simulink model "MX_Gateway"  (Simulink 9.0)
settings: Solver=FixedStepDiscrete  StartTime=0.0  StopTime=100  FixedStep=0.01  RelTol=1e-3
values from neighbouring files (symbols this model uses):
  ACTIVE = Bobcat.Parameter; CreateDefine( ACTIVE                         ,1                          ,'Define' ,'defines.h' )    [defines.m]
  ACTIVE_MODE_ONE = Bobcat.Parameter; CreateDefine( ACTIVE_MODE_ONE                ,19                         ,'Define' ,'defines.h' )    [defines.m]
  AUTHENTICATED = Bobcat.Parameter; CreateDefine( AUTHENTICATED                  ,1                          ,'Define' ,'defines.h' )    [defines.m]
  AUX_EMEA_CONTROLS = Bobcat.Parameter; CreateDefine( AUX_EMEA_CONTROLS  ,1   ,'Define' ,'defines.h' )    [enums.m]
  AUX_FLOW_HIGH = Bobcat.Parameter; CreateDefine( AUX_FLOW_HIGH           ,4  ,'Define' ,'defines.h' )    [enums.m]
  AUX_FLOW_INACTIVE = Bobcat.Parameter; CreateDefine( AUX_FLOW_INACTIVE       ,0  ,'Define' ,'defines.h' )    [enums.m]
  AUX_FLOW_LOW = Bobcat.Parameter; CreateDefine( AUX_FLOW_LOW            ,1  ,'Define' ,'defines.h' )    [enums.m]
  AUX_FLOW_MEDIUM = Bobcat.Parameter; CreateDefine( AUX_FLOW_MEDIUM         ,2  ,'Define' ,'defines.h' )    [enums.m]
  AUX_FLOW_STANDARD = Bobcat.Parameter; CreateDefine( AUX_FLOW_STANDARD       ,3  ,'Define' ,'defines.h' )    [enums.m]
  AUX_NON_FUNCTIONAL = Bobcat.Parameter; CreateDefine( AUX_NON_FUNCTIONAL ,255 ,'Define' ,'defines.h' )    [enums.m]
  BASE_ACTIVE = Bobcat.Parameter; CreateDefine( BASE_ACTIVE                    ,253                        ,'Define' ,'defines.h' )    [defines.m]
  BASE_DETENT = Bobcat.Parameter; CreateDefine( BASE_DETENT                    ,1                          ,'Define' ,'defines.h' )    [defines.m]
  BEEP_NONE = Bobcat.Parameter; CreateDefine( BEEP_NONE                      ,255                        ,'Define' ,'defines.h' )    [defines.m]
  CD_AIR_FILTER_DEBOUNCE_NORMAL = Bobcat.Parameter; CreateAliasCD( CD_AIR_FILTER_DEBOUNCE_NORMAL                        ,uint16(500)                ,'ConstantDataPointer' ,'CONSTANT_DATA' ,'AIR_FILTER_DEBOUNCE_NORMAL')    [cdata.m]    (also in hc12_cdata.m; m4_cdata.m)
  CD_AIR_FILTER_DEBOUNCE_NOT_CONNECTED = Bobcat.Parameter; CreateAliasCD( CD_AIR_FILTER_DEBOUNCE_NOT_CONNECTED                 ,uint16(3000)               ,'ConstantDataPointer' ,'CONSTANT_DATA' ,'AIR_FILTER_DEBOUNCE_NOT_CONNECTED')    [cdata.m]    (also in hc12_cdata.m; m4_cdata.m)
  CD_AIR_FILTER_DEBOUNCE_PLUGGED = Bobcat.Parameter; CreateAliasCD( CD_AIR_FILTER_DEBOUNCE_PLUGGED                       ,uint16(3000)               ,'ConstantDataPointer' ,'CONSTANT_DATA' ,'AIR_FILTER_DEBOUNCE_PLUGGED')    [cdata.m]    (also in hc12_cdata.m; m4_cdata.m)
  CD_AIR_FILTER_FUNCTION = Bobcat.Parameter; CreateAliasCD( CD_AIR_FILTER_FUNCTION                               ,uint8(255)                 ,'ConstantDataPointer' ,'CONSTANT_DATA' ,'AIR_FILTER_FUNCTION')    [cdata.m]    (also in hc12_cdata.m; m4_cdata.m)
  CD_AIR_FILTER_PLUGGED_WEIGHT = Bobcat.Parameter; CreateAliasCD( CD_AIR_FILTER_PLUGGED_WEIGHT                         ,uint8(2)                   ,'ConstantDataPointer' ,'CONSTANT_DATA' ,'AIR_FILTER_PLUGGED_WEIGHT')    [cdata.m]    (also in hc12_cdata.m; m4_cdata.m)
  CD_AIR_FILTER_UNPLUGGED_WEIGHT = Bobcat.Parameter; CreateAliasCD( CD_AIR_FILTER_UNPLUGGED_WEIGHT                       ,uint8(1)                   ,'ConstantDataPointer' ,'CONSTANT_DATA' ,'AIR_FILTER_UNPLUGGED_WEIGHT')    [cdata.m]    (also in hc12_cdata.m; m4_cdata.m)
  CD_AUTOIDLE_ARMBAR_DELAY = Bobcat.Parameter; CreateAliasCD( CD_AUTOIDLE_ARMBAR_DELAY                             ,uint8(2)                   ,'ConstantDataPointer' ,'CONSTANT_DATA' ,'AUTOIDLE_ARMBAR_DELAY')    [cdata.m]    (also in hc12_cdata.m; m4_cdata.m)
  CD_AUTOIDLE_FUNCTION = Bobcat.Parameter; CreateAliasCD( CD_AUTOIDLE_FUNCTION                                 ,uint8(255)                 ,'ConstantDataPointer' ,'CONSTANT_DATA' ,'AUTOIDLE_FUNCTION')    [cdata.m]    (also in hc12_cdata.m; m4_cdata.m)
  CD_AUTOIDLE_IDLE_DELAY = Bobcat.Parameter; CreateAliasCD( CD_AUTOIDLE_IDLE_DELAY                               ,uint8(40)                  ,'ConstantDataPointer' ,'CONSTANT_DATA' ,'AUTOIDLE_IDLE_DELAY')    [cdata.m]    (also in hc12_cdata.m; m4_cdata.m)
  CD_AUTOIDLE_LOW_IDLE_START = Bobcat.Parameter; CreateAliasCD( CD_AUTOIDLE_LOW_IDLE_START                           ,uint8(255)                 ,'ConstantDataPointer' ,'CONSTANT_DATA' ,'AUTOIDLE_LOW_IDLE_START')    [cdata.m]    (also in hc12_cdata.m; m4_cdata.m)
  CD_AUTOIDLE_PRESSURE_OFF = Bobcat.Parameter; CreateAliasCD( CD_AUTOIDLE_PRESSURE_OFF                             ,uint16(300)                ,'ConstantDataPointer' ,'CONSTANT_DATA' ,'AUTOIDLE_PRESSURE_OFF')    [cdata.m]    (also in hc12_cdata.m; m4_cdata.m)
  CD_AUTOIDLE_PRESSURE_ON = Bobcat.Parameter; CreateAliasCD( CD_AUTOIDLE_PRESSURE_ON                              ,uint16(270)                ,'ConstantDataPointer' ,'CONSTANT_DATA' ,'AUTOIDLE_PRESSURE_ON')    [cdata.m]    (also in hc12_cdata.m; m4_cdata.m)
  CD_AUTOSHIFT_FUNCTION = Bobcat.Parameter; CreateAliasCD( CD_AUTOSHIFT_FUNCTION                                ,uint8(255)                 ,'ConstantDataPointer' ,'CONSTANT_DATA' ,'AUTOSHIFT_FUNCTION')    [cdata.m]    (also in hc12_cdata.m; m4_cdata.m)
  CD_AUTOSHIFT_PHIGH1 = Bobcat.Parameter; CreateAliasCD( CD_AUTOSHIFT_PHIGH1                                  ,uint16(205)                ,'ConstantDataPointer' ,'CONSTANT_DATA' ,'AUTOSHIFT_PHIGH1')    [cdata.m]    (also in hc12_cdata.m; m4_cdata.m)
  CD_AUTOSHIFT_PHIGH2 = Bobcat.Parameter; CreateAliasCD( CD_AUTOSHIFT_PHIGH2                                  ,uint16(193)                ,'ConstantDataPointer' ,'CONSTANT_DATA' ,'AUTOSHIFT_PHIGH2')    [cdata.m]    (also in hc12_cdata.m; m4_cdata.m)
  CD_AUTOSHIFT_PHIGH_TEMP1 = Bobcat.Parameter; CreateAliasCD( CD_AUTOSHIFT_PHIGH_TEMP1                             ,int16(70)                  ,'ConstantDataPointer' ,'CONSTANT_DATA' ,'AUTOSHIFT_PHIGH_TEMP1')    [cdata.m]    (also in hc12_cdata.m; m4_cdata.m)
  CD_AUTOSHIFT_PHIGH_TEMP2 = Bobcat.Parameter; CreateAliasCD( CD_AUTOSHIFT_PHIGH_TEMP2                             ,int16(160)                 ,'ConstantDataPointer' ,'CONSTANT_DATA' ,'AUTOSHIFT_PHIGH_TEMP2')    [cdata.m]    (also in hc12_cdata.m; m4_cdata.m)
  CD_AUTOSHIFT_PLOW1 = Bobcat.Parameter; CreateAliasCD( CD_AUTOSHIFT_PLOW1                                   ,uint16(130)                ,'ConstantDataPointer' ,'CONSTANT_DATA' ,'AUTOSHIFT_PLOW1')    [cdata.m]    (also in hc12_cdata.m; m4_cdata.m)
  CD_AUTOSHIFT_PLOW2 = Bobcat.Parameter; CreateAliasCD( CD_AUTOSHIFT_PLOW2                                   ,uint16(100)                ,'ConstantDataPointer' ,'CONSTANT_DATA' ,'AUTOSHIFT_PLOW2')    [cdata.m]    (also in hc12_cdata.m; m4_cdata.m)
  CD_AUTOSHIFT_PLOW_TEMP1 = Bobcat.Parameter; CreateAliasCD( CD_AUTOSHIFT_PLOW_TEMP1                              ,int16(20)                  ,'ConstantDataPointer' ,'CONSTANT_DATA' ,'AUTOSHIFT_PLOW_TEMP1')    [cdata.m]    (also in hc12_cdata.m; m4_cdata.m)
  CD_AUTOSHIFT_PLOW_TEMP2 = Bobcat.Parameter; CreateAliasCD( CD_AUTOSHIFT_PLOW_TEMP2                              ,int16(80)                  ,'ConstantDataPointer' ,'CONSTANT_DATA' ,'AUTOSHIFT_PLOW_TEMP2')    [cdata.m]    (also in hc12_cdata.m; m4_cdata.m)
  CD_AUTOSHIFT_TRAVEL_DELAY = Bobcat.Parameter; CreateAliasCD( CD_AUTOSHIFT_TRAVEL_DELAY                            ,uint8(32)                  ,'ConstantDataPointer' ,'CONSTANT_DATA' ,'AUTOSHIFT_TRAVEL_DELAY')    [cdata.m]    (also in hc12_cdata.m; m4_cdata.m)
  CD_AUX2_CRACKPOINT_CURRENT = Bobcat.Parameter; CreateAliasCD( CD_AUX2_CRACKPOINT_CURRENT                           ,fi(0,0,16,12,'hex','0c28') ,'ConstantDataPointer' ,'CONSTANT_DATA' ,'AUX2_CRACKPOINT_CURRENT')    [cdata.m]    (also in hc12_cdata.m; m4_cdata.m)
  CD_AUX2_FLOW_LOW_FUNCTION = Bobcat.Parameter; CreateAliasCD( CD_AUX2_FLOW_LOW_FUNCTION                            ,uint8(255)                 ,'ConstantDataPointer' ,'CONSTANT_DATA' ,'AUX2_FLOW_LOW_FUNCTION')    [cdata.m]    (also in hc12_cdata.m; m4_cdata.m)
  CD_AUX2_FLOW_MED_FUNCTION = Bobcat.Parameter; CreateAliasCD( CD_AUX2_FLOW_MED_FUNCTION                            ,uint8(255)                 ,'ConstantDataPointer' ,'CONSTANT_DATA' ,'AUX2_FLOW_MED_FUNCTION')    [cdata.m]    (also in hc12_cdata.m; m4_cdata.m)
  CD_AUX2_HI_FLOW_PERCENT = Bobcat.Parameter; CreateAliasCD( CD_AUX2_HI_FLOW_PERCENT                              ,fi(0,0,16,16,'hex','ffff') ,'ConstantDataPointer' ,'CONSTANT_DATA' ,'AUX2_HI_FLOW_PERCENT')    [cdata.m]    (also in hc12_cdata.m; m4_cdata.m)
  CD_AUX2_LOW_FLOW_PERCENT = Bobcat.Parameter; CreateAliasCD( CD_AUX2_LOW_FLOW_PERCENT                             ,fi(0,0,16,16,'hex','7333') ,'ConstantDataPointer' ,'CONSTANT_DATA' ,'AUX2_LOW_FLOW_PERCENT')    [cdata.m]    (also in hc12_cdata.m; m4_cdata.m)
  CD_AUX2_MAX_FLOW_CURRENT = Bobcat.Parameter; CreateAliasCD( CD_AUX2_MAX_FLOW_CURRENT                             ,fi(0,0,16,12,'hex','14cc') ,'ConstantDataPointer' ,'CONSTANT_DATA' ,'AUX2_MAX_FLOW_CURRENT')    [cdata.m]    (also in hc12_cdata.m; m4_cdata.m)
  CD_AUX2_MED_FLOW_PERCENT = Bobcat.Parameter; CreateAliasCD( CD_AUX2_MED_FLOW_PERCENT                             ,fi(0,0,16,16,'hex','9999') ,'ConstantDataPointer' ,'CONSTANT_DATA' ,'AUX2_MED_FLOW_PERCENT')    [cdata.m]    (also in hc12_cdata.m; m4_cdata.m)
  CD_AUX_CRACKPOINT_CURRENT = Bobcat.Parameter; CreateAliasCD( CD_AUX_CRACKPOINT_CURRENT                            ,fi(0,0,16,12,'hex','0000') ,'ConstantDataPointer' ,'CONSTANT_DATA' ,'AUX_CRACKPOINT_CURRENT')    [cdata.m]    (also in hc12_cdata.m; m4_cdata.m)
  CD_AUX_DETENT_FLASH_TIME = Bobcat.Parameter; CreateAliasCD( CD_AUX_DETENT_FLASH_TIME                             ,uint16(72)                 ,'ConstantDataPointer' ,'CONSTANT_DATA' ,'AUX_DETENT_FLASH_TIME')    [cdata.m]    (also in hc12_cdata.m; m4_cdata.m)
  CD_AUX_DETENT_HOLD_TIME = Bobcat.Parameter; CreateAliasCD( CD_AUX_DETENT_HOLD_TIME                              ,uint16(100)                ,'ConstantDataPointer' ,'CONSTANT_DATA' ,'AUX_DETENT_HOLD_TIME')    [cdata.m]    (also in hc12_cdata.m; m4_cdata.m)
  CD_AUX_FULL_ON_AUX1 = Bobcat.Parameter; CreateAliasCD( CD_AUX_FULL_ON_AUX1                                  ,fi(0,0,16,12,'hex','10cd') ,'ConstantDataPointer' ,'CONSTANT_DATA' ,'AUX_FULL_ON_AUX1')    [cdata.m]    (also in hc12_cdata.m; m4_cdata.m)
  CD_AUX_FULL_ON_AUX2 = Bobcat.Parameter; CreateAliasCD( CD_AUX_FULL_ON_AUX2                                  ,fi(0,0,16,12,'hex','139d') ,'ConstantDataPointer' ,'CONSTANT_DATA' ,'AUX_FULL_ON_AUX2')    [cdata.m]    (also in hc12_cdata.m; m4_cdata.m)
  CD_AUX_FULL_ON_AUX3 = Bobcat.Parameter; CreateAliasCD( CD_AUX_FULL_ON_AUX3                                  ,fi(0,0,16,12,'hex','17d7') ,'ConstantDataPointer' ,'CONSTANT_DATA' ,'AUX_FULL_ON_AUX3')    [cdata.m]    (also in hc12_cdata.m; m4_cdata.m)
  CD_AUX_FUNCTION = Bobcat.Parameter; CreateAliasCD( CD_AUX_FUNCTION                                      ,uint8(0)                   ,'ConstantDataPointer' ,'CONSTANT_DATA' ,'AUX_FUNCTION')    [cdata.m]    (also in hc12_cdata.m; m4_cdata.m)
  CD_AUX_RATE_LIMIT_CRACKPOINT = Bobcat.Parameter; CreateAliasCD( CD_AUX_RATE_LIMIT_CRACKPOINT                         ,fi(0,0,16,12,'hex','0800') ,'ConstantDataPointer' ,'CONSTANT_DATA' ,'AUX_RATE_LIMIT_CRACKPOINT')    [cdata.m]    (also in hc12_cdata.m; m4_cdata.m)
  CD_AUX_RATE_LIMIT_CRACKPOINT_RATE = Bobcat.Parameter; CreateAliasCD( CD_AUX_RATE_LIMIT_CRACKPOINT_RATE                    ,fi(0,1,32,12,'int',102400) ,'ConstantDataPointer' ,'CONSTANT_DATA' ,'AUX_RATE_LIMIT_CRACKPOINT_RATE')    [cdata.m]    (also in hc12_cdata.m; m4_cdata.m)
  CD_AUX_RATE_LIMIT_FUNCTION = Bobcat.Parameter; CreateAliasCD( CD_AUX_RATE_LIMIT_FUNCTION                           ,uint8(255)                 ,'ConstantDataPointer' ,'CONSTANT_DATA' ,'AUX_RATE_LIMIT_FUNCTION')    [cdata.m]    (also in hc12_cdata.m; m4_cdata.m)
  CD_AUX_RATE_LIMIT_MAX_ENG_SPD = Bobcat.Parameter; CreateAliasCD( CD_AUX_RATE_LIMIT_MAX_ENG_SPD                        ,uint16(1600)               ,'ConstantDataPointer' ,'CONSTANT_DATA' ,'AUX_RATE_LIMIT_MAX_ENG_SPD')    [cdata.m]    (also in hc12_cdata.m; m4_cdata.m)
  CD_AUX_RATE_LIMIT_MIN_ENG_SPD = Bobcat.Parameter; CreateAliasCD( CD_AUX_RATE_LIMIT_MIN_ENG_SPD                        ,uint16(100)                ,'ConstantDataPointer' ,'CONSTANT_DATA' ,'AUX_RATE_LIMIT_MIN_ENG_SPD')    [cdata.m]    (also in hc12_cdata.m; m4_cdata.m)
  CD_AUX_RATE_LIMIT_NORMAL_RATE = Bobcat.Parameter; CreateAliasCD( CD_AUX_RATE_LIMIT_NORMAL_RATE                        ,fi(0,1,32,12,'int',2048)   ,'ConstantDataPointer' ,'CONSTANT_DATA' ,'AUX_RATE_LIMIT_NORMAL_RATE')    [cdata.m]    (also in hc12_cdata.m; m4_cdata.m)
  CD_AUXPOT_CAL_DEADBAND_HIGH = Bobcat.Parameter; CreateAliasCD( CD_AUXPOT_CAL_DEADBAND_HIGH                          ,uint16(574)                ,'ConstantDataPointer' ,'CONSTANT_DATA' ,'AUXPOT_CAL_DEADBAND_HIGH')    [cdata.m]    (also in hc12_cdata.m; m4_cdata.m)
  CD_AUXPOT_CAL_DEADBAND_LOW = Bobcat.Parameter; CreateAliasCD( CD_AUXPOT_CAL_DEADBAND_LOW                           ,uint16(424)                ,'ConstantDataPointer' ,'CONSTANT_DATA' ,'AUXPOT_CAL_DEADBAND_LOW')    [cdata.m]    (also in hc12_cdata.m; m4_cdata.m)
  CD_AUXPOT_CENTER_RATIO = Bobcat.Parameter; CreateAliasCD( CD_AUXPOT_CENTER_RATIO                               ,uint16(500)                ,'ConstantDataPointer' ,'CONSTANT_DATA' ,'AUXPOT_CENTER_RATIO')    [cdata.m]    (also in hc12_cdata.m; m4_cdata.m)
  CD_AUXPOT_DEADBAND = Bobcat.Parameter; CreateAliasCD( CD_AUXPOT_DEADBAND                                   ,uint8(38)                  ,'ConstantDataPointer' ,'CONSTANT_DATA' ,'AUXPOT_DEADBAND')    [cdata.m]    (also in hc12_cdata.m; m4_cdata.m)
  CD_AUXPOT_FUNCTION = Bobcat.Parameter; CreateAliasCD( CD_AUXPOT_FUNCTION                                   ,uint8(0)                   ,'ConstantDataPointer' ,'CONSTANT_DATA' ,'AUXPOT_FUNCTION')    [cdata.m]    (also in hc12_cdata.m; m4_cdata.m)
  CD_AUXPOT_RATIO_GAIN = Bobcat.Parameter; CreateAliasCD( CD_AUXPOT_RATIO_GAIN                                 ,uint16(1000)               ,'ConstantDataPointer' ,'CONSTANT_DATA' ,'AUXPOT_RATIO_GAIN')    [cdata.m]    (also in hc12_cdata.m; m4_cdata.m)
  CD_CAN_THROTTLE_FUNCTION = Bobcat.Parameter; CreateAliasCD( CD_CAN_THROTTLE_FUNCTION                             ,uint8(255)                 ,'ConstantDataPointer' ,'CONSTANT_DATA' ,'CAN_THROTTLE_FUNCTION')    [cdata.m]    (also in hc12_cdata.m; m4_cdata.m)
  CD_CAN_THROTTLE_REVERSE = Bobcat.Parameter; CreateAliasCD( CD_CAN_THROTTLE_REVERSE                              ,uint8(255)                 ,'ConstantDataPointer' ,'CONSTANT_DATA' ,'CAN_THROTTLE_REVERSE')    [cdata.m]    (also in hc12_cdata.m; m4_cdata.m)
  CD_CONSOLE_DEBOUNCE_ABSENT = Bobcat.Parameter; CreateAliasCD( CD_CONSOLE_DEBOUNCE_ABSENT                           ,uint16(12)                 ,'ConstantDataPointer' ,'CONSTANT_DATA' ,'CONSOLE_DEBOUNCE_ABSENT')    [cdata.m]    (also in hc12_cdata.m; m4_cdata.m)
  CD_CONSOLE_DEBOUNCE_OORH = Bobcat.Parameter; CreateAliasCD( CD_CONSOLE_DEBOUNCE_OORH                             ,uint16(12)                 ,'ConstantDataPointer' ,'CONSTANT_DATA' ,'CONSOLE_DEBOUNCE_OORH')    [cdata.m]    (also in hc12_cdata.m; m4_cdata.m)
  CD_CONSOLE_DEBOUNCE_OORL = Bobcat.Parameter; CreateAliasCD( CD_CONSOLE_DEBOUNCE_OORL                             ,uint16(12)                 ,'ConstantDataPointer' ,'CONSTANT_DATA' ,'CONSOLE_DEBOUNCE_OORL')    [cdata.m]    (also in hc12_cdata.m; m4_cdata.m)
  CD_CONSOLE_DEBOUNCE_PRESENT = Bobcat.Parameter; CreateAliasCD( CD_CONSOLE_DEBOUNCE_PRESENT                          ,uint16(12)                 ,'ConstantDataPointer' ,'CONSTANT_DATA' ,'CONSOLE_DEBOUNCE_PRESENT')    [cdata.m]    (also in hc12_cdata.m; m4_cdata.m)
  CD_CONSOLE_FUNCTION = Bobcat.Parameter; CreateAliasCD( CD_CONSOLE_FUNCTION                                  ,uint8(0)                   ,'ConstantDataPointer' ,'CONSTANT_DATA' ,'CONSOLE_FUNCTION')    [cdata.m]    (also in hc12_cdata.m; m4_cdata.m)
  CD_CONSOLE_OORH = Bobcat.Parameter; CreateAliasCD( CD_CONSOLE_OORH                                      ,uint16(740)                ,'ConstantDataPointer' ,'CONSTANT_DATA' ,'CONSOLE_OORH')    [cdata.m]    (also in hc12_cdata.m; m4_cdata.m)
  CD_CONSOLE_OORL = Bobcat.Parameter; CreateAliasCD( CD_CONSOLE_OORL                                      ,uint16(10)                 ,'ConstantDataPointer' ,'CONSTANT_DATA' ,'CONSOLE_OORL')    [cdata.m]    (also in hc12_cdata.m; m4_cdata.m)
  CD_CONSOLE_PRESENT_HIGH = Bobcat.Parameter; CreateAliasCD( CD_CONSOLE_PRESENT_HIGH                              ,uint16(515)                ,'ConstantDataPointer' ,'CONSTANT_DATA' ,'CONSOLE_PRESENT_HIGH')    [cdata.m]    (also in hc12_cdata.m; m4_cdata.m)
  CD_CONSOLE_PRESENT_LOW = Bobcat.Parameter; CreateAliasCD( CD_CONSOLE_PRESENT_LOW                               ,uint16(375)                ,'ConstantDataPointer' ,'CONSTANT_DATA' ,'CONSOLE_PRESENT_LOW')    [cdata.m]    (also in hc12_cdata.m; m4_cdata.m)
  CD_DELUXE_GEN5_5_FUNCTION = Bobcat.Parameter; CreateAliasCD( CD_DELUXE_GEN5_5_FUNCTION                            ,uint8(255)                 ,'ConstantDataPointer' ,'CONSTANT_DATA' ,'DELUXE_GEN5_5_FUNCTION')    [cdata.m]    (also in hc12_cdata.m; m4_cdata.m)
  CD_DELUXE_GEN5_FUNCTION = Bobcat.Parameter; CreateAliasCD( CD_DELUXE_GEN5_FUNCTION                              ,uint8(255)                 ,'ConstantDataPointer' ,'CONSTANT_DATA' ,'DELUXE_GEN5_FUNCTION')    [cdata.m]    (also in hc12_cdata.m; m4_cdata.m)
  CD_DERATE_ENGINE_SPEED_DEBOUNCE_HIGH = Bobcat.Parameter; CreateAliasCD( CD_DERATE_ENGINE_SPEED_DEBOUNCE_HIGH                 ,uint16(200)                ,'ConstantDataPointer' ,'CONSTANT_DATA' ,'DERATE_ENGINE_SPEED_DEBOUNCE_HIGH')    [cdata.m]    (also in hc12_cdata.m; m4_cdata.m)
  CD_DERATE_ENGINE_SPEED_DEBOUNCE_SHUTDOWN = Bobcat.Parameter; CreateAliasCD( CD_DERATE_ENGINE_SPEED_DEBOUNCE_SHUTDOWN             ,uint16(1000)               ,'ConstantDataPointer' ,'CONSTANT_DATA' ,'DERATE_ENGINE_SPEED_DEBOUNCE_SHUTDOWN')    [cdata.m]    (also in hc12_cdata.m; m4_cdata.m)
  CD_DERATE_ENGINE_SPEED_HIGH_OFFSET = Bobcat.Parameter; CreateAliasCD( CD_DERATE_ENGINE_SPEED_HIGH_OFFSET                   ,uint16(200)                ,'ConstantDataPointer' ,'CONSTANT_DATA' ,'DERATE_ENGINE_SPEED_HIGH_OFFSET')    [cdata.m]    (also in hc12_cdata.m; m4_cdata.m)
  CD_DERATE_METHOD = Bobcat.Parameter; CreateAliasCD( CD_DERATE_METHOD                                     ,uint8(0)                   ,'ConstantDataPointer' ,'CONSTANT_DATA' ,'DERATE_METHOD')    [cdata.m]    (also in hc12_cdata.m; m4_cdata.m)
  CD_DUMP_VALVE_FUNCTION = Bobcat.Parameter; CreateAliasCD( CD_DUMP_VALVE_FUNCTION                               ,uint8(255)                 ,'ConstantDataPointer' ,'CONSTANT_DATA' ,'DUMP_VALVE_FUNCTION')    [cdata.m]    (also in hc12_cdata.m; m4_cdata.m)
  CD_DUMP_VALVE_ON_CURRENT = Bobcat.Parameter; CreateAliasCD( CD_DUMP_VALVE_ON_CURRENT                             ,fi(0,0,16,12,'hex','ffff') ,'ConstantDataPointer' ,'CONSTANT_DATA' ,'DUMP_VALVE_ON_CURRENT')    [cdata.m]    (also in hc12_cdata.m; m4_cdata.m)
  CD_ECO_MODE_THROTTLE_REDUCTION = Bobcat.Parameter; CreateAliasCD( CD_ECO_MODE_THROTTLE_REDUCTION                       ,uint16(850)                ,'ConstantDataPointer' ,'CONSTANT_DATA' ,'ECO_MODE_THROTTLE_REDUCTION')    [cdata.m]    (also in hc12_cdata.m; m4_cdata.m)
  CD_ECT_FILTER_GAIN = Bobcat.Parameter; CreateAliasCD( CD_ECT_FILTER_GAIN                                   ,uint8(6)                   ,'ConstantDataPointer' ,'CONSTANT_DATA' ,'ECT_FILTER_GAIN')    [cdata.m]    (also in hc12_cdata.m; m4_cdata.m)
  CD_ECU_FUNCTION = Bobcat.Parameter; CreateAliasCD( CD_ECU_FUNCTION                                      ,uint8(255)                 ,'ConstantDataPointer' ,'CONSTANT_DATA' ,'ECU_FUNCTION')    [cdata.m]    (also in hc12_cdata.m; m4_cdata.m)
  CD_ECU_HOLD_DEBOUNCE_ERROR_ON = Bobcat.Parameter; CreateAliasCD( CD_ECU_HOLD_DEBOUNCE_ERROR_ON                        ,uint16(20)                 ,'ConstantDataPointer' ,'CONSTANT_DATA' ,'ECU_HOLD_DEBOUNCE_ERROR_ON')    [cdata.m]    (also in hc12_cdata.m; m4_cdata.m)
  CD_EMEA_CONTROLS_HOLD_TIME = Bobcat.Parameter; CreateAliasCD( CD_EMEA_CONTROLS_HOLD_TIME                           ,uint16(30)                 ,'ConstantDataPointer' ,'CONSTANT_DATA' ,'EMEA_CONTROLS_HOLD_TIME')    [cdata.m]    (also in hc12_cdata.m; m4_cdata.m)
  CD_EMEA_CONTROLS_OPTION_A = Bobcat.Parameter; CreateAliasCD( CD_EMEA_CONTROLS_OPTION_A                            ,uint8(255)                 ,'ConstantDataPointer' ,'CONSTANT_DATA' ,'EMEA_CONTROLS_OPTION_A')    [cdata.m]    (also in hc12_cdata.m; m4_cdata.m)
  CD_EMEA_CONTROLS_OPTION_C = Bobcat.Parameter; CreateAliasCD( CD_EMEA_CONTROLS_OPTION_C                            ,uint8(255)                 ,'ConstantDataPointer' ,'CONSTANT_DATA' ,'EMEA_CONTROLS_OPTION_C')    [cdata.m]    (also in hc12_cdata.m; m4_cdata.m)
  CD_EMEA_CONTROLS_REVERSE = Bobcat.Parameter; CreateAliasCD( CD_EMEA_CONTROLS_REVERSE                             ,uint8(255)                 ,'ConstantDataPointer' ,'CONSTANT_DATA' ,'EMEA_CONTROLS_REVERSE')    [cdata.m]    (also in hc12_cdata.m; m4_cdata.m)
  CD_EMEA_ELECTRIC_VERSION = Bobcat.Parameter; CreateAliasCD( CD_EMEA_ELECTRIC_VERSION                             ,uint8(0)                   ,'ConstantDataPointer' ,'CONSTANT_DATA' ,'EMEA_ELECTRIC_VERSION')    [cdata.m]    (also in hc12_cdata.m; m4_cdata.m)
  CD_EMEA_POT_CAL_DEADBAND_HIGH = Bobcat.Parameter; CreateAliasCD( CD_EMEA_POT_CAL_DEADBAND_HIGH                        ,uint16(574)                ,'ConstantDataPointer' ,'CONSTANT_DATA' ,'EMEA_POT_CAL_DEADBAND_HIGH')    [cdata.m]    (also in hc12_cdata.m; m4_cdata.m)
  CD_EMEA_POT_CAL_DEADBAND_LOW = Bobcat.Parameter; CreateAliasCD( CD_EMEA_POT_CAL_DEADBAND_LOW                         ,uint16(424)                ,'ConstantDataPointer' ,'CONSTANT_DATA' ,'EMEA_POT_CAL_DEADBAND_LOW')    [cdata.m]    (also in hc12_cdata.m; m4_cdata.m)
  CD_ENGINE_RPM_FACTOR = Bobcat.Parameter; CreateAliasCD( CD_ENGINE_RPM_FACTOR                                 ,uint8(35)                  ,'ConstantDataPointer' ,'CONSTANT_DATA' ,'ENGINE_RPM_FACTOR')    [cdata.m]    (also in hc12_cdata.m; m4_cdata.m)
  CD_ENGINE_SPEED_FILTER_FAST_VALUE = Bobcat.Parameter; CreateAliasCD( CD_ENGINE_SPEED_FILTER_FAST_VALUE                    ,uint8(1)                   ,'ConstantDataPointer' ,'CONSTANT_DATA' ,'ENGINE_SPEED_FILTER_FAST_VALUE')    [cdata.m]    (also in hc12_cdata.m; m4_cdata.m)
  CD_ENGINE_SPEED_FILTER_SLOW_VALUE = Bobcat.Parameter; CreateAliasCD( CD_ENGINE_SPEED_FILTER_SLOW_VALUE                    ,uint8(4)                   ,'ConstantDataPointer' ,'CONSTANT_DATA' ,'ENGINE_SPEED_FILTER_SLOW_VALUE')    [cdata.m]    (also in hc12_cdata.m; m4_cdata.m)
  CD_ENGINE_SPEED_RUNNING = Bobcat.Parameter; CreateAliasCD( CD_ENGINE_SPEED_RUNNING                              ,uint16(800)                ,'ConstantDataPointer' ,'CONSTANT_DATA' ,'ENGINE_SPEED_RUNNING')    [cdata.m]    (also in hc12_cdata.m; m4_cdata.m)
  CD_ENGINE_START_DELAY_DEBOUNCE_RPM_NO_SIGNAL = Bobcat.Parameter; CreateAliasCD( CD_ENGINE_START_DELAY_DEBOUNCE_RPM_NO_SIGNAL         ,uint16(0)                  ,'ConstantDataPointer' ,'CONSTANT_DATA' ,'ENGINE_START_DELAY_DEBOUNCE_RPM_NO_SIGNAL')    [cdata.m]    (also in hc12_cdata.m; m4_cdata.m)
  CD_ENGINE_START_DELAY_OFF_TIME = Bobcat.Parameter; CreateAliasCD( CD_ENGINE_START_DELAY_OFF_TIME                       ,uint16(0)                  ,'ConstantDataPointer' ,'CONSTANT_DATA' ,'ENGINE_START_DELAY_OFF_TIME')    [cdata.m]    (also in hc12_cdata.m; m4_cdata.m)
  CD_ENGINE_START_DELAY_RPM_NO_SIGNAL = Bobcat.Parameter; CreateAliasCD( CD_ENGINE_START_DELAY_RPM_NO_SIGNAL                  ,uint16(0)                  ,'ConstantDataPointer' ,'CONSTANT_DATA' ,'ENGINE_START_DELAY_RPM_NO_SIGNAL')    [cdata.m]    (also in hc12_cdata.m; m4_cdata.m)
  CD_EOP_DEBOUNCE_EXTREMELY_LOW = Bobcat.Parameter; CreateAliasCD( CD_EOP_DEBOUNCE_EXTREMELY_LOW                        ,uint16(50)                 ,'ConstantDataPointer' ,'CONSTANT_DATA' ,'EOP_DEBOUNCE_EXTREMELY_LOW')    [cdata.m]    (also in hc12_cdata.m; m4_cdata.m)
  CD_EOP_DEBOUNCE_NORMAL = Bobcat.Parameter; CreateAliasCD( CD_EOP_DEBOUNCE_NORMAL                               ,uint16(50)                 ,'ConstantDataPointer' ,'CONSTANT_DATA' ,'EOP_DEBOUNCE_NORMAL')    [cdata.m]    (also in hc12_cdata.m; m4_cdata.m)
  CD_EOP_DEBOUNCE_SHUTDOWN = Bobcat.Parameter; CreateAliasCD( CD_EOP_DEBOUNCE_SHUTDOWN                             ,uint16(100)                ,'ConstantDataPointer' ,'CONSTANT_DATA' ,'EOP_DEBOUNCE_SHUTDOWN')    [cdata.m]    (also in hc12_cdata.m; m4_cdata.m)
  CD_EOP_FILTER_VALUE = Bobcat.Parameter; CreateAliasCD( CD_EOP_FILTER_VALUE                                  ,uint8(3)                   ,'ConstantDataPointer' ,'CONSTANT_DATA' ,'EOP_FILTER_VALUE')    [cdata.m]    (also in hc12_cdata.m; m4_cdata.m)
  CD_EOP_FUNCTION = Bobcat.Parameter; CreateAliasCD( CD_EOP_FUNCTION                                      ,uint8(1)                   ,'ConstantDataPointer' ,'CONSTANT_DATA' ,'EOP_FUNCTION')    [cdata.m]    (also in hc12_cdata.m; m4_cdata.m)
  CD_EOP_SWITCH_GAUGE_VALUE = Bobcat.Parameter; CreateAliasCD( CD_EOP_SWITCH_GAUGE_VALUE                            ,uint16(125)                ,'ConstantDataPointer' ,'CONSTANT_DATA' ,'EOP_SWITCH_GAUGE_VALUE')    [cdata.m]    (also in hc12_cdata.m; m4_cdata.m)
  CD_EOP_SWITCH_LOW = Bobcat.Parameter; CreateAliasCD( CD_EOP_SWITCH_LOW                                    ,uint16(50)                 ,'ConstantDataPointer' ,'CONSTANT_DATA' ,'EOP_SWITCH_LOW')    [cdata.m]    (also in hc12_cdata.m; m4_cdata.m)
  CD_EOP_SWITCH_PRESSURE_VALUE = Bobcat.Parameter; CreateAliasCD( CD_EOP_SWITCH_PRESSURE_VALUE                         ,uint16(40)                 ,'ConstantDataPointer' ,'CONSTANT_DATA' ,'EOP_SWITCH_PRESSURE_VALUE')    [cdata.m]    (also in hc12_cdata.m; m4_cdata.m)
  CD_EOT_DEBOUNCE_EXTREMELY_LOW = Bobcat.Parameter; CreateAliasCD( CD_EOT_DEBOUNCE_EXTREMELY_LOW                        ,uint16(2)                  ,'ConstantDataPointer' ,'CONSTANT_DATA' ,'EOT_DEBOUNCE_EXTREMELY_LOW')    [cdata.m]    (also in hc12_cdata.m; m4_cdata.m)
  CD_EOT_DEBOUNCE_LOW = Bobcat.Parameter; CreateAliasCD( CD_EOT_DEBOUNCE_LOW                                  ,uint16(2)                  ,'ConstantDataPointer' ,'CONSTANT_DATA' ,'EOT_DEBOUNCE_LOW')    [cdata.m]    (also in hc12_cdata.m; m4_cdata.m)
  CD_EOT_DEBOUNCE_NORMAL = Bobcat.Parameter; CreateAliasCD( CD_EOT_DEBOUNCE_NORMAL                               ,uint16(2)                  ,'ConstantDataPointer' ,'CONSTANT_DATA' ,'EOT_DEBOUNCE_NORMAL')    [cdata.m]    (also in hc12_cdata.m; m4_cdata.m)
  CD_EOT_EXTREMELY_LOW = Bobcat.Parameter; CreateAliasCD( CD_EOT_EXTREMELY_LOW                                 ,uint16(81)                 ,'ConstantDataPointer' ,'CONSTANT_DATA' ,'EOT_EXTREMELY_LOW')    [cdata.m]    (also in hc12_cdata.m; m4_cdata.m)
  CD_EOT_FUNCTION = Bobcat.Parameter; CreateAliasCD( CD_EOT_FUNCTION                                      ,uint8(255)                 ,'ConstantDataPointer' ,'CONSTANT_DATA' ,'EOT_FUNCTION')    [cdata.m]    (also in hc12_cdata.m; m4_cdata.m)
  CD_EOT_LOW = Bobcat.Parameter; CreateAliasCD( CD_EOT_LOW                                           ,uint16(90)                 ,'ConstantDataPointer' ,'CONSTANT_DATA' ,'EOT_LOW')    [cdata.m]    (also in hc12_cdata.m; m4_cdata.m)
  CD_EOT_TEMPERATURE_HYSTERESIS = Bobcat.Parameter; CreateAliasCD( CD_EOT_TEMPERATURE_HYSTERESIS                        ,uint16(2)                  ,'ConstantDataPointer' ,'CONSTANT_DATA' ,'EOT_TEMPERATURE_HYSTERESIS')    [cdata.m]    (also in hc12_cdata.m; m4_cdata.m)
  CD_FAST_TRAVEL_FUNCTION = Bobcat.Parameter; CreateAliasCD( CD_FAST_TRAVEL_FUNCTION                              ,uint8(255)                 ,'ConstantDataPointer' ,'CONSTANT_DATA' ,'FAST_TRAVEL_FUNCTION')    [cdata.m]    (also in hc12_cdata.m; m4_cdata.m)
  CD_FAST_TRAVEL_THROTTLE_DELAY = Bobcat.Parameter; CreateAliasCD( CD_FAST_TRAVEL_THROTTLE_DELAY                        ,uint16(10)                 ,'ConstantDataPointer' ,'CONSTANT_DATA' ,'FAST_TRAVEL_THROTTLE_DELAY')    [cdata.m]    (also in hc12_cdata.m; m4_cdata.m)
  CD_FAST_TRAVEL_THROTTLE_LIMIT = Bobcat.Parameter; CreateAliasCD( CD_FAST_TRAVEL_THROTTLE_LIMIT                        ,uint16(1000)               ,'ConstantDataPointer' ,'CONSTANT_DATA' ,'FAST_TRAVEL_THROTTLE_LIMIT')    [cdata.m]    (also in hc12_cdata.m; m4_cdata.m)
  CD_FLYWHEEL_TEETH = Bobcat.Parameter; CreateAliasCD( CD_FLYWHEEL_TEETH                                    ,uint8(83)                  ,'ConstantDataPointer' ,'CONSTANT_DATA' ,'FLYWHEEL_TEETH')    [cdata.m]    (also in hc12_cdata.m; m4_cdata.m)
  CD_FUEL_LEVEL_FILTER_FAST_VALUE = Bobcat.Parameter; CreateAliasCD( CD_FUEL_LEVEL_FILTER_FAST_VALUE                      ,uint8(5)                   ,'ConstantDataPointer' ,'CONSTANT_DATA' ,'FUEL_LEVEL_FILTER_FAST_VALUE')    [cdata.m]    (also in hc12_cdata.m; m4_cdata.m)
  CD_FUEL_LEVEL_FILTER_SLOW_VALUE = Bobcat.Parameter; CreateAliasCD( CD_FUEL_LEVEL_FILTER_SLOW_VALUE                      ,uint8(10)                  ,'ConstantDataPointer' ,'CONSTANT_DATA' ,'FUEL_LEVEL_FILTER_SLOW_VALUE')    [cdata.m]    (also in hc12_cdata.m; m4_cdata.m)
  CD_FUEL_PRIMARY_FEEDBACK_FUNCTION = Bobcat.Parameter; CreateAliasCD( CD_FUEL_PRIMARY_FEEDBACK_FUNCTION                    ,uint8(0)                   ,'ConstantDataPointer' ,'CONSTANT_DATA' ,'FUEL_PRIMARY_FEEDBACK_FUNCTION')    [cdata.m]    (also in hc12_cdata.m; m4_cdata.m)
  ... (+246 more)
undefined symbols (not defined in the file or in its folder): sigbuilder_block
undefined symbols (not defined in the file): CD_FUEL_RESOLUTION_TIMER
libraries: simulink x186, MX_Library x107
(part 44 of 48)

== stateflow chart MX_Gtwy_Control/PanelInterface/mod_HydraulicIcon/HydraulicIcon ==
  settings: executeAtInitialization=1
  state INACTIVE
    en:icon_Hydraulic = INACTIVE;
  state ERROR
    en:icon_Hydraulic = ERROR_FLASH;
  state ACTIVE
    en:icon_Hydraulic = ACTIVE;
  (default) -> INACTIVE
  INACTIVE -> ERROR [order 1] : [(state_HOT == EXTREMELY_HIGH)...
      | || (state_HOT == SHUTDOWN)]
  ERROR -> INACTIVE : [(state_HOT == NORMAL) || (state_HOT == HIGH) || (state_HOT == COLD)]
  INACTIVE -> ACTIVE [order 2] : [(state_HOT == HIGH)...
      | || (state_HOT == OORL)...
      | || (state_HOT == OORH)]
  ACTIVE -> INACTIVE [order 1] : [(state_HOT == NORMAL) || (state_HOT == COLD)]
  ACTIVE -> ERROR [order 2] : [ (state_HOT == EXTREMELY_HIGH)...
      | || (state_HOT == SHUTDOWN)]
  data: icon_Hydraulic output uint8; state_HOT input uint8; ACTIVE parameter uint8; COLD parameter uint8; EXTREMELY_HIGH parameter uint8; HIGH parameter uint8; INACTIVE parameter uint8; NORMAL parameter uint8; SHUTDOWN parameter uint8; OORL parameter uint8; OORH parameter uint8; ERROR_FLASH parameter uint8

== matlab function MX_Gtwy_Control/PanelInterface/mod_JobClock1Icon/Truth_Table ==
  data: u input uint8; y output uint8
function y = fcn(u)

aVarTruthTableCondition_1 = false;


% Job clock 1 is active

aVarTruthTableCondition_1 = logical(u == 6);

if (aVarTruthTableCondition_1)
    aFcnTruthTableAction_1();
else % Default
    aFcnTruthTableAction_2();
end

function aFcnTruthTableAction_1()

% Turn on icon.

y = uint8(1);

function aFcnTruthTableAction_2()

% Turn off icon.

y = uint8(0);

== matlab function MX_Gtwy_Control/PanelInterface/mod_JobClock2Icon/Truth_Table ==
  data: u input uint8; y output uint8
function y = fcn(u)

aVarTruthTableCondition_1 = false;


% Job clock 2 is active

aVarTruthTableCondition_1 = logical(u == 7);

if (aVarTruthTableCondition_1)
    aFcnTruthTableAction_1();
else % Default
    aFcnTruthTableAction_2();
end

function aFcnTruthTableAction_1()

% Turn on icon.

y = uint8(1);

function aFcnTruthTableAction_2()

% Turn off icon.

y = uint8(0);

== stateflow chart MX_Gtwy_Control/PanelInterface/mod_RPMIcon/RPM_ICON ==
  settings: executeAtInitialization=1
  state ERROR
    en:icon_RPM = ERROR_FLASH;
  state INACTIVE
    en:icon_RPM = INACTIVE;
  state ACTIVE
    en:icon_RPM = ACTIVE;
  (default) -> INACTIVE
  INACTIVE -> ERROR [order 1] : [(state_EngineSpeed != NORMAL)]
  ERROR -> INACTIVE : [(state_EngineSpeed == NORMAL)]
  INACTIVE -> ACTIVE [order 2] : [(state_InfoPrimary == DISPLAY_ENGINE_RPM)]
  ACTIVE -> INACTIVE [order 1] : [state_InfoPrimary != DISPLAY_ENGINE_RPM]
  ACTIVE -> ERROR [order 2] : [state_EngineSpeed != NORMAL]
  data: state_InfoPrimary input uint8; state_EngineSpeed input uint8; icon_RPM output uint8; ACTIVE parameter uint8; DISPLAY_ENGINE_RPM parameter uint8; INACTIVE parameter uint8; NORMAL parameter uint8; ERROR_FLASH parameter uint8

== stateflow chart MX_Gtwy_Control/PanelInterface/mod_LockoutIcon/LockoutIcons ==
  state ERROR
    en:icon_Lockout = ERROR_FLASH;
  state INACTIVE
    en:icon_Lockout = INACTIVE;
  state ACTIVE
    en:icon_Lockout = ACTIVE;
  (default) -> INACTIVE
  INACTIVE -> ERROR [order 1] : [(state_Console == OORH)...
      | || (state_Console == OORL)...
      | || (state_ConsoleTest == FAILURE)...
      | || (state_HydEnable == SHORT_TO_BATTERY)...
      | || (state_HydEnable == SHORT_TO_GROUND)...
      | || (state_HydEnable == OPEN_CIRCUIT)...
      | || (state_HydEnable == OVERCURRENT)...
      | || (state_HydBypass== SHORT_TO_BATTERY)...
      | || (state_HydBypass == SHORT_TO_GROUND)...
      | || (state_HydBypass == OPEN_CIRCUIT)...
      | || (state_HydBypass == OVERCURRENT)]
  ERROR -> INACTIVE : [(state_Console != OORH)...
      | && (state_Console != OORL)...
      | && (state_ConsoleTest != FAILURE)...
      | && (state_HydEnable != SHORT_TO_BATTERY)...
      | && (state_HydEnable != SHORT_TO_GROUND)...
      | && (state_HydEnable != OPEN_CIRCUIT)...
      | && (state_HydEnable != OVERCURRENT)...
      | && (state_HydBypass != SHORT_TO_BATTERY)...
      | && (state_HydBypass != SHORT_TO_GROUND)...
      | && (state_HydBypass != OPEN_CIRCUIT)...
      | && (state_HydBypass != OVERCURRENT)]
  INACTIVE -> ACTIVE [order 2] : [(state_HydEnable == INACTIVE)]
  ACTIVE -> INACTIVE [order 1] : [(state_HydEnable == ACTIVE)]
  ACTIVE -> ERROR [order 2] : [(state_Console == OORH)...
      | || (state_Console == OORL)...
      | || (state_ConsoleTest == FAILURE)...
      | || (state_HydEnable == SHORT_TO_BATTERY)...
      | || (state_HydEnable == SHORT_TO_GROUND)...
      | || (state_HydEnable == OPEN_CIRCUIT)...
      | || (state_HydEnable == OVERCURRENT)...
      | || (state_HydBypass == SHORT_TO_BATTERY)...
      | || (state_HydBypass == SHORT_TO_GROUND)...
      | || (state_HydBypass == OPEN_CIRCUIT)...
      | || (state_HydBypass == OVERCURRENT)]
  data: state_Console input uint8; icon_Lockout output uint8; ACTIVE parameter uint8; INACTIVE parameter uint8; ERROR_FLASH parameter uint8; state_ConsoleTest input uint8; FAILURE parameter uint8; OORH parameter uint8; OORL parameter uint8; state_HydEnable input uint8; OPEN_CIRCUIT parameter uint8; SHORT_TO_BATTERY parameter uint8; SHORT_TO_GROUND parameter uint8; OVERCURRENT parameter uint8; state_HydBypass input uint8

== stateflow chart MX_Gtwy_Control/PanelInterface/mod_SeatBeltIcon/Chart ==
  settings: executeAtInitialization=1
  state ACTIVE
    en:icon_Seatbelt = ACTIVE;
    en:debounceCount = 0;
    du:debounceCount++;
  state INACTIVE
    en:icon_Seatbelt = INACTIVE;
  (default) -> ACTIVE
  ACTIVE -> INACTIVE : [(debounceCount >= 450)]
  data: icon_Seatbelt output uint8; debounceCount local uint16 =0; ACTIVE parameter uint8; INACTIVE parameter uint8

== matlab function MX_Gtwy_Control/PanelInterface/mod_ServiceClockIcon/Truth_Table ==
  data: state_InfoSecondary input uint8; icon_Service output uint8; state_AirFilter input uint8; state_HCFilter1 input uint8; PLUGGED parameter uint8; state_InfoPrimary input uint8; NOT_CONNECTED parameter uint8; ee_DeluxeG5 data_store_memory double; ACTIVE parameter uint8; CD_DELUXE_GEN5_5_FUNCTION parameter uint8; NON_FUNCTIONAL parameter uint8
function icon_Service = fcn(state_InfoSecondary, state_AirFilter, state_HCFilter1, PLUGGED, state_InfoPrimary, NOT_CONNECTED, ACTIVE, CD_DELUXE_GEN5_5_FUNCTION, NON_FUNCTIONAL)

aVarTruthTableCondition_1 = false;
aVarTruthTableCondition_2 = false;
aVarTruthTableCondition_3 = false;
aVarTruthTableCondition_4 = false;
aVarTruthTableCondition_5 = false;
aVarTruthTableCondition_6 = false;
aVarTruthTableCondition_7 = false;


% Error Codes are active

aVarTruthTableCondition_1 = logical(state_InfoPrimary == 5);


aVarTruthTableCondition_2 = logical(state_HCFilter1 == PLUGGED);

% Air Filter Plugged

aVarTruthTableCondition_3 = logical(state_AirFilter == PLUGGED);

% Filter not connected

aVarTruthTableCondition_4 = logical(state_HCFilter1 == NOT_CONNECTED);


aVarTruthTableCondition_5 = logical(state_AirFilter == NOT_CONNECTED);

% Service Clock is in alarm

aVarTruthTableCondition_6 = logical(state_InfoSecondary == 11 && ee_DeluxeG5 ~= ACTIVE && CD_DELUXE_GEN5_5_FUNCTION == NON_FUNCTIONAL);

% Service Clock is active

aVarTruthTableCondition_7 = logical(state_InfoPrimary == 4);

if (aVarTruthTableCondition_7)
    aFcnTruthTableAction_1();
elseif (aVarTruthTableCondition_3)
    aFcnTruthTableAction_3();
elseif (aVarTruthTableCondition_2)
    aFcnTruthTableAction_3();
elseif (aVarTruthTableCondition_1)
    aFcnTruthTableAction_1();
elseif (aVarTruthTableCondition_4)
    aFcnTruthTableAction_3();
elseif (aVarTruthTableCondition_5)
    aFcnTruthTableAction_3();
elseif (aVarTruthTableCondition_6)
    aFcnTruthTableAction_3();
else % Default
    aFcnTruthTableAction_2();
end

function aFcnTruthTableAction_1()

% Turn on icon.

icon_Service = uint8(1);

function aFcnTruthTableAction_2()

% Turn off icon.

icon_Service = uint8(0);

function aFcnTruthTableAction_3()

% Flash Icon

icon_Service = uint8(2);

== stateflow chart MX_Gtwy_Control/System/Throttle_Command/Throttle_Command ==
  state Throttle_Actuator
    state Normal
      en:
      cmd_Throttle = WORD_UNAVAIL;
    state AutoIdle
      en:
      cmd_Throttle = CD_LOW_IDLE_COMMAND;
    (default) -> Throttle_Actuator.Normal
    Throttle_Actuator.AutoIdle -> Throttle_Actuator.Normal : [cmd_AutoIdle != ACTIVE]
    Throttle_Actuator.Normal -> Throttle_Actuator.AutoIdle : [cmd_AutoIdle == ACTIVE]
  state G2_Engine
    state WAIT_FOR_EOT
      en:cmd_Throttle = 0;
      en:debounceTC = 0;
      en:state_TC = INACTIVE;
    state TC_PROTECTION
      state COLD
        en:state_TC = LOW;
        en:cmd_Throttle = CD_TC_PROTECT_THROTTLE_LOW;
        en:debounceTC = 0;
        du:debounceTC++;
        state CHECK_THROTTLE
          en:state_TC = LOW;
          en:Throttle_old = pos_Throttle;
          du:Throttle_diff = pos_Throttle - Throttle_old;
          du:Throttle_diff = abs(Throttle_diff);
        state THROTTLE_MOVED
          en: state_TC = EVENT;
          en: debounceEvent = 0;
          en:state_Buzzer = TRIPLE_SHORT_BEEP;
          en:Throttle_diff = 0;
          du: debounceEvent++;
        (default) -> G2_Engine.TC_PROTECTION.COLD.CHECK_THROTTLE
        G2_Engine.TC_PROTECTION.COLD.CHECK_THROTTLE -> G2_Engine.TC_PROTECTION.COLD.THROTTLE_MOVED : [(Throttle_diff >= CD_TC_PROTECT_THROTTLE_DIFFERENCE)]
        G2_Engine.TC_PROTECTION.COLD.THROTTLE_MOVED -> G2_Engine.TC_PROTECTION.COLD.CHECK_THROTTLE : [(debounceEvent >= CD_TC_PROTECT_THROTTLE_DEBOUNCE_LOW_EVENT)...
            | && (CD_TC_PROTECT_THROTTLE_DEBOUNCE_LOW_EVENT != UNLIMITED)]
      state TIMER_RESET
      state EXTREMELY_COLD
        en:state_TC = EXTREMELY_LOW;
        en:cmd_Throttle = CD_TC_PROTECT_THROTTLE_EXT_LOW;
        state CHECK_THROTTLE
          en:state_TC = EXTREMELY_LOW;
          en:Throttle_old = pos_Throttle;
          du:Throttle_diff = pos_Throttle - Throttle_old;
          du:Throttle_diff = abs(Throttle_diff);
        state THROTTLE_MOVED
          en: state_TC = EVENT;
          en: debounceEvent = 0;
          en:state_Buzzer = TRIPLE_SHORT_BEEP;
          en:Throttle_diff = 0;
          du: debounceEvent++;
        (default) -> G2_Engine.TC_PROTECTION.EXTREMELY_COLD.CHECK_THROTTLE
        G2_Engine.TC_PROTECTION.EXTREMELY_COLD.CHECK_THROTTLE -> G2_Engine.TC_PROTECTION.EXTREMELY_COLD.THROTTLE_MOVED : [(Throttle_diff >= CD_TC_PROTECT_THROTTLE_DIFFERENCE)]
        G2_Engine.TC_PROTECTION.EXTREMELY_COLD.THROTTLE_MOVED -> G2_Engine.TC_PROTECTION.EXTREMELY_COLD.CHECK_THROTTLE : [(debounceEvent >= CD_TC_PROTECT_THROTTLE_DEBOUNCE_EXT_LOW_EVENT)...
            | && (CD_TC_PROTECT_THROTTLE_DEBOUNCE_EXT_LOW_EVENT != UNLIMITED)]
      (default) -> COLD
      COLD -> TIMER_RESET [order 2] : [state_Engine != ENGINE_RUNNING]
      TIMER_RESET -> COLD : [state_Engine == ENGINE_RUNNING]
      EXTREMELY_COLD -> COLD : [(state_EOT != EXTREMELY_LOW)]
      COLD -> EXTREMELY_COLD [order 1] : [(state_EOT == EXTREMELY_LOW)]
    state Normal
      state Normal_Throttle
        en:
        cmd_Throttle = pos_Throttle;
        du:
        cmd_Throttle = pos_Throttle;
      state AutoIdle
        en:
        cmd_Throttle = CD_LOW_IDLE_COMMAND;
      (default) -> Normal_Throttle
      Normal_Throttle -> G2_Engine.Normal.AutoIdle : [cmd_AutoIdle == ACTIVE]
      G2_Engine.Normal.AutoIdle -> Normal_Throttle : [cmd_AutoIdle != ACTIVE]
    state WAIT_FOR_THROTTLE_MOVEMENT
      en:state_TC = NORMAL;
      en:Throttle_old = pos_Throttle;
      en:state_Buzzer = DOUBLE_SHORT_BEEP;
      en:Throttle_diff = 0;
      du:Throttle_diff = pos_Throttle - Throttle_old;
      du:Throttle_diff = abs(Throttle_diff);
    (default) -> WAIT_FOR_EOT
    WAIT_FOR_EOT -> TC_PROTECTION [order 2] : [((state_EOT == LOW) || (state_EOT == EXTREMELY_LOW))...
        | && (CD_TC_PROTECT_FUNCTION != NON_FUNCTIONAL)...
        | && (state_Engine == ENGINE_RUNNING)]
    WAIT_FOR_EOT -> G2_Engine.Normal [order 1] : [(state_EOT == NORMAL) && (state_Engine == ENGINE_RUNNING)...
        | || (CD_TC_PROTECT_FUNCTION == NON_FUNCTIONAL)]
    TC_PROTECTION -> WAIT_FOR_THROTTLE_MOVEMENT : [((flt_EOP >= CD_TC_PROTECT_EOP_LOW) && (state_ecuEOP == NORMAL))...
        | && ((debounceTC >= CD_TC_PROTECT_DEBOUNCE_ENABLE) && (CD_TC_PROTECT_DEBOUNCE_ENABLE != UNLIMITED))]
    WAIT_FOR_THROTTLE_MOVEMENT -> G2_Engine.Normal : [(Throttle_diff >= CD_TC_PROTECT_THROTTLE_DIFFERENCE)]
  state CAN_Throttle_Actuator
    state Normal
      en:
      cmd_Throttle = cmd_CANThrottle;
      du:
      cmd_Throttle = cmd_CANThrottle;
    state AutoIdle
      en:
      cmd_Throttle = ee_ThrottleCalExtended;
    state AutoIdle_Reversed
      en:
      cmd_Throttle = ee_ThrottleCalRetracted;
    (default) -> CAN_Throttle_Actuator.Normal
    CAN_Throttle_Actuator.Normal -> CAN_Throttle_Actuator.AutoIdle [order 1] : [cmd_AutoIdle == ACTIVE...
        | && CD_CAN_THROTTLE_REVERSE == NON_FUNCTIONAL]
    CAN_Throttle_Actuator.AutoIdle -> CAN_Throttle_Actuator.Normal : [cmd_AutoIdle != ACTIVE]
    CAN_Throttle_Actuator.Normal -> AutoIdle_Reversed [order 2] : [cmd_AutoIdle == ACTIVE...
        | && CD_CAN_THROTTLE_REVERSE != NON_FUNCTIONAL]
    AutoIdle_Reversed -> CAN_Throttle_Actuator.Normal : [cmd_AutoIdle != ACTIVE]
  state Invalid
    en:
    cmd_Throttle = CD_LOW_IDLE_COMMAND;
  (default) -> (junction 5598)
  (junction 5598) -> Throttle_Actuator [order 1] : [CD_THROTTLE_PRIMARY_FUNCTION == NON_FUNCTIONAL]
  (junction 5598) -> (junction 5599) [order 2]
  (junction 5599) -> G2_Engine [order 1] : [CD_ECU_FUNCTION != NON_FUNCTIONAL]
  (junction 5599) -> (junction 5600) [order 2]
  (junction 5600) -> CAN_Throttle_Actuator [order 1] : [CD_CAN_THROTTLE_FUNCTION != NON_FUNCTIONAL]
  (junction 5600) -> Invalid [order 2]
  data: ACTIVE parameter uint8; CD_LOW_IDLE_COMMAND parameter uint16; cmd_AutoIdle input uint8; cmd_Throttle output uint16; cmd_CANThrottle input uint16; pos_Throttle input uint16; CD_THROTTLE_PRIMARY_FUNCTION parameter uint8; CD_ECU_FUNCTION parameter uint8; CD_CAN_THROTTLE_FUNCTION parameter uint8; NON_FUNCTIONAL parameter uint8; WORD_UNAVAIL parameter uint16; ee_ThrottleCalExtended data_store_memory double; CD_TC_PROTECT_DEBOUNCE_ENABLE parameter uint16; CD_TC_PROTECT_FUNCTION parameter uint8; CD_TC_PROTECT_THROTTLE_DEBOUNCE_EXT_LOW_EVENT parameter uint16; CD_TC_PROTECT_THROTTLE_DEBOUNCE_LOW_EVENT parameter uint16; CD_TC_PROTECT_THROTTLE_DIFFERENCE parameter uint16; CD_TC_PROTECT_THROTTLE_EXT_LOW parameter uint16; CD_TC_PROTECT_THROTTLE_LOW parameter uint16; DOUBLE_SHORT_BEEP parameter uint8; EVENT parameter uint8; EXTREMELY_LOW parameter uint8; INACTIVE parameter uint8; LOW parameter uint8; NORMAL parameter uint8; TRIPLE_SHORT_BEEP parameter uint8; Throttle_diff local int16; Throttle_old local uint16; UNLIMITED parameter uint16; debounceEvent local uint16; debounceTC local uint16; state_Buzzer data_store_memory double; state_EOT input uint8; state_TC output uint8; state_Engine input uint8; ENGINE_RUNNING parameter uint8; state_ecuEOP input uint8; flt_EOP input uint16; CD_TC_PROTECT_EOP_LOW parameter uint16; CD_CAN_THROTTLE_REVERSE parameter uint8; ee_ThrottleCalRetracted data_store_memory double

== stateflow chart MX_Gtwy_Control/System/EMEA Control Mode/Right Handle Switch ==
  state Default_InactiveCheck
    en:
    request = 0;
  state Normal
    state DebounceActive
    state Inactive
      en:
      request = 0;
    state Active
      en:
      request = 1;
      du:
      request = 0;
    (default) -> Inactive
    Inactive -> DebounceActive : [din_Sw == ACTIVE]
    DebounceActive -> Inactive [order 2] : [din_Sw == INACTIVE]
    Active -> Inactive : [din_Sw == INACTIVE]
    DebounceActive -> Active [order 1] : [after(CD_EMEA_CONTROLS_HOLD_TIME,tick)]
  (default) -> Default_InactiveCheck
  Default_InactiveCheck -> Normal : [din_Sw == INACTIVE...
      | && state_CommSecondary == NORMAL]
  Normal -> Default_InactiveCheck : [state_CommSecondary != NORMAL]
  data: din_Sw input boolean; INACTIVE parameter uint8; ACTIVE parameter uint8; request output boolean; CD_EMEA_CONTROLS_HOLD_TIME parameter uint16; state_CommSecondary input uint8; NORMAL parameter uint8
